simulink model "wlan_phy_rx_pmd"  (Simulink 7.8)
settings: Solver=VariableStepDiscrete  SolverMode=SingleTasking  StartTime=0.0  StopTime=rx_sim.sim_time  RelTol=1e-3
PostLoadFcn:
  | wlan_phy_rx_init
InitFcn:
  | wlan_phy_rx_init
StartFcn:
  | wlan_phy_rx_init
values from neighbouring files (symbols this model uses):
  barker_seq20 = 0.95 * circshift(barker_seq20, [0 4]) ./ max(abs(barker_seq20))    [wlan_phy_rx_init.m]
  bit_scrambler_lfsr_bytes = sum(bit_scrambler_lfsr_bytes .* repmat(2.^[0:7], 127, 1),2)    [wlan_phy_rx_init.m]
  longCorr_coef_i = [3*real(longCorr_coef)]    [wlan_phy_rx_init.m]
  longCorr_coef_q = [3*imag(longCorr_coef)]    [wlan_phy_rx_init.m]
  MAX_CP_LEN = 32    [wlan_phy_rx_init.m]
  MAX_NCBPS = NCBPS_11n_64QAM    (= 312)    [wlan_phy_rx_init.m]
  MAX_NUM_BYTES = 2^16-1    (= 65535)    [wlan_phy_rx_init.m]
  MAX_NUM_SC = 64    [wlan_phy_rx_init.m]
  MAX_NUM_SYMS = 600    [wlan_phy_rx_init.m]
  NDBPS_11a_BPSK12 = 24    [wlan_phy_rx_init.m]
  preFFT_sampBuff_numSamps = 4*MAX_NUM_SC    (= 256)    [wlan_phy_rx_init.m]
  rx_sim = struct()    [wlan_phy_rx_init.m]
  scr_ind_rev = zeros(1,128)    [wlan_phy_rx_init.m]
undefined symbols (not defined in the file or in its folder): gcbh, mcs_rom_11ag, mcs_rom_11n, wlan_rx_deinterleave_rom_gen, xlBlockLoadCallback, xlGetNormalizedPeriod, xlipgetpartsetting
libraries: xbsIndex_r4 x4943, xbsEDKLib_r4 x1
(part 9 of 28)

== system wlan_phy_rx_pmd/Chan Est & EQ/Debug Outputs/4x Buffer ==
  I : Inport
  Q : Inport  Port=2
  Vin : Inport  Port=3
  14LSB : xbsIndex_r4/Slice  nbits=14  boolean_output=off  mode=Lower Bit Location + Width  bit1=0  base1=MSB of Input  bit0=0  base0=LSB of Input  dbl_ovrd=off  block_type=slice
  14MSB : xbsIndex_r4/Slice  nbits=14  boolean_output=off  mode=Upper Bit Location + Width  bit1=0  base1=MSB of Input  bit0=0  base0=LSB of Input  dbl_ovrd=off  block_type=slice
  Concat : xbsIndex_r4/Concat  num_inputs=2  dbl_ovrd=off  block_type=concat
  Constant3 : xbsIndex_r4/Constant  const=1  gui_display_data_type=Boolean  arith_type=Boolean  n_bits=5  bin_pt=0  preci_type=Single  exp_width=8  frac_width=24  explicit_period=on  period=4  equ=P=C  opselect=C  inp2=PCIN>>17  opr=+  inp1=P  carry=CIN  dbl_ovrd=off  block_type=constant
  FIFO : xbsIndex_r4/FIFO  mem_type=Block RAM  performance_options=Standard_FIFO  infoedit1=Available only for V4, V5, V6, V7, K7, A7 and Zynq devices.  embedded_registers=off  depth=64  percent_nbits=1  rst=off  en=off  use_dcount=off  use_percent_full_port=off  use_almost_empty=off  almost_empty_thresh=2  use_almost_full=off  almost_full_thresh=14  dbl_ovrd=off  explicit_period=off  block_type=fifo
  Reinterpret : xbsIndex_r4/Reinterpret  force_arith_type=on  arith_type=Unsigned  force_bin_pt=on  bin_pt=0  block_type=reinterpret
  Reinterpret1 : xbsIndex_r4/Reinterpret  force_arith_type=on  arith_type=Unsigned  force_bin_pt=on  bin_pt=0  block_type=reinterpret
  Reinterpret2 : xbsIndex_r4/Reinterpret  force_arith_type=on  arith_type="Signed  (2's comp)"  force_bin_pt=on  bin_pt=13  block_type=reinterpret
  Reinterpret3 : xbsIndex_r4/Reinterpret  force_arith_type=on  arith_type="Signed  (2's comp)"  force_bin_pt=on  bin_pt=13  block_type=reinterpret
  Up Sample : xbsIndex_r4/Up Sample  sample_ratio=4  copy_samples=on  en=off  latency=0  dbl_ovrd=off  block_type=usamp
  Up Sample1 : xbsIndex_r4/Up Sample  sample_ratio=4  copy_samples=on  en=off  latency=0  dbl_ovrd=off  block_type=usamp
  " I" : Outport
  " Q" : Outport  Port=2
  #Vout : Outport  Port=3
  wires:
  Constant3/1 -> FIFO/3
  Concat/1 -> FIFO/1
  I/1 -> Reinterpret/1
  14MSB/1 -> Reinterpret2/1
  Q/1 -> Reinterpret1/1
  14LSB/1 -> Reinterpret3/1
  Vin/1 -> FIFO/2
  FIFO/2 -> #Vout/1
  Reinterpret/1 -> Concat/1
  Reinterpret1/1 -> Concat/2
  Reinterpret2/1 -> Up Sample/1
  Reinterpret3/1 -> Up Sample1/1
  Up Sample/1 -> " I"/1
  Up Sample1/1 -> " Q"/1
  FIFO/1 -> 14LSB/1, 14MSB/1

== system wlan_phy_rx_pmd/Chan Est & EQ/Equalizer, Pilot Processing & PHY Mode Det/Mag ==
  I : Inport
  Q : Inport  Port=2
  AddSub : xbsIndex_r4/AddSub  mode=Addition  use_carryin=off  use_carryout=off  en=off  latency=4  precision=Full  arith_type=Unsigned  n_bits=16  bin_pt=14  quantization=Truncate  overflow=Wrap  dbl_ovrd=off  use_behavioral_HDL=off  hw_selection=DSP48  pipelined=off  block_type=addsub
  Mult : xbsIndex_r4/Mult  precision=Full  arith_type="Signed  (2's comp)"  n_bits=16  bin_pt=14  quantization=Truncate  overflow=Wrap  en=off  latency=2  dbl_ovrd=off  use_behavioral_HDL=off  opt=Speed  use_embedded=on  optimum_pipeline=off  pipeline=on  use_rpm=on  placement_style=Triangular  block_type=mult
  Mult1 : xbsIndex_r4/Mult  precision=Full  arith_type="Signed  (2's comp)"  n_bits=16  bin_pt=14  quantization=Truncate  overflow=Wrap  en=off  latency=2  dbl_ovrd=off  use_behavioral_HDL=off  opt=Speed  use_embedded=on  optimum_pipeline=off  pipeline=on  use_rpm=on  placement_style=Triangular  block_type=mult
  Register1 : xbsIndex_r4/Register  init=0  rst=off  en=off  dbl_ovrd=off  block_type=register
  Register2 : xbsIndex_r4/Register  init=0  rst=off  en=off  dbl_ovrd=off  block_type=register
  Register3 : xbsIndex_r4/Register  init=0  rst=off  en=off  dbl_ovrd=off  block_type=register
  M : Outport
  wires:
  I/1 -> Mult/1, Mult/2
  Q/1 -> Mult1/1, Mult1/2
  Mult/1 -> Register2/1
  Mult1/1 -> Register1/1
  AddSub/1 -> Register3/1
  Register2/1 -> AddSub/1
  Register1/1 -> AddSub/2
  Register3/1 -> M/1

== system wlan_phy_rx_pmd/Chan Est & EQ/Equalizer, Pilot Processing & PHY Mode Det/PHY Mode Det ==
  note: OFDM Symbols in 11a/g:
      | 0: LTF (BPSK)
      | 1: LTF (BPSK)
      | 2: SIG (BPSK)
      | 3-N: Data
  note: PHY Mode
      | 001: 11a/g
      | 010: 11n
      | 100: 11ac
  note: OFDM Symbols in 11n SISO:
      | 0: L-LTF (BPSK)
      | 1: L-LTF (BPSK)
      | 2: L-SIG (BPSK)
      | 3: HT-SIG1 (QBPSK)
      | 4: HT-SIG2 (QBPSK)
      | 5: HT-STF
      | 6: HT-LFT1
      | 7-N: Data
  note: OFDM Symbols in 11ac SISO:
      | 0: L-LTF (BPSK)
      | 1: L-LTF (BPSK)
      | 2: L-SIG (BPSK)
      | 3: VHT-SIGA1 (BPSK)
      | 4: VHT-SIGA2 (QBPSK)
      | 5: VHT-STF
      | 6: VHT-LFT1
      | 7: VHT-SIGB
      | 8-N: Data
  note: Assert rates here to avoid
      | rate ambiguity when these
      | signals are fed back to earlier
      | blocks in the pipeline
  note: Mark all Rx as 11a/g when 11n mode
      | is disabled.
  x_I : Inport
  x_Q : Inport  Port=2
  x_valid : Inport  Port=3
  Sym ind : Inport  Port=4
  " 11ac" : xbsIndex_r4/Register  init=0  rst=on  en=on  dbl_ovrd=off  block_type=register
  " 11ag" : xbsIndex_r4/Register  init=1  rst=on  en=on  dbl_ovrd=off  block_type=register
  " 11n" : xbsIndex_r4/Register  init=0  rst=on  en=on  dbl_ovrd=off  block_type=register
  Absolute : xbsIndex_r4/Absolute  precision=User Defined  arith_type="Signed  (2's comp)"  n_bits=8  bin_pt=7  quantization=Truncate  overflow=Saturate  en=off  latency=1  dbl_ovrd=off  block_type=abs
  Absolute1 : xbsIndex_r4/Absolute  precision=User Defined  arith_type="Signed  (2's comp)"  n_bits=8  bin_pt=7  quantization=Truncate  overflow=Saturate  en=off  latency=1  dbl_ovrd=off  block_type=abs
  Assert : xbsIndex_r4/Assert  assert_type=off  type_source=Explicitly  gui_display_data_type=Fixed-point  arith_type="Signed  (2's comp)"  n_bits=16  bin_pt=14  preci_type=Single  exp_width=8  frac_width=24  assert_rate=on  rate_source=Explicitly  period=1  output_port=on  block_type=assert
  Assert1 : xbsIndex_r4/Assert  assert_type=off  type_source=Explicitly  gui_display_data_type=Fixed-point  arith_type="Signed  (2's comp)"  n_bits=16  bin_pt=14  preci_type=Single  exp_width=8  frac_width=24  assert_rate=on  rate_source=Explicitly  period=1  output_port=on  block_type=assert
  Assert2 : xbsIndex_r4/Assert  assert_type=off  type_source=Explicitly  gui_display_data_type=Fixed-point  arith_type="Signed  (2's comp)"  n_bits=16  bin_pt=14  preci_type=Single  exp_width=8  frac_width=24  assert_rate=on  rate_source=Explicitly  period=1  output_port=on  block_type=assert
  Concat : xbsIndex_r4/Concat  num_inputs=2  dbl_ovrd=off  block_type=concat
  Concat1 : xbsIndex_r4/Concat  num_inputs=3  dbl_ovrd=off  block_type=concat
  Concat2 : xbsIndex_r4/Concat  num_inputs=3  dbl_ovrd=off  block_type=concat
  Constant1 : xbsIndex_r4/Constant  const=0  gui_display_data_type=Fixed-point  arith_type=Unsigned  n_bits=2  bin_pt=0  preci_type=Single  exp_width=8  frac_width=24  explicit_period=off  period=1  equ=P=C  opselect=C  inp2=PCIN>>17  opr=+  inp1=P  carry=CIN  dbl_ovrd=off  block_type=constant
  Constant3 : xbsIndex_r4/Constant  const=3  gui_display_data_type=Fixed-point  arith_type=Unsigned  n_bits=2  bin_pt=0  preci_type=Single  exp_width=8  frac_width=24  explicit_period=off  period=1  equ=P=C  opselect=C  inp2=PCIN>>17  opr=+  inp1=P  carry=CIN  dbl_ovrd=off  block_type=constant
  Constant4 : xbsIndex_r4/Constant  const=4  gui_display_data_type=Fixed-point  arith_type=Unsigned  n_bits=3  bin_pt=0  preci_type=Single  exp_width=8  frac_width=24  explicit_period=off  period=1  equ=P=C  opselect=C  inp2=PCIN>>17  opr=+  inp1=P  carry=CIN  dbl_ovrd=off  block_type=constant
  Constant5 : xbsIndex_r4/Constant  const=1  gui_display_data_type=Fixed-point  arith_type=Unsigned  n_bits=2  bin_pt=0  preci_type=Single  exp_width=8  frac_width=24  explicit_period=off  period=1  equ=P=C  opselect=C  inp2=PCIN>>17  opr=+  inp1=P  carry=CIN  dbl_ovrd=off  block_type=constant
  Constant7 : xbsIndex_r4/Constant  const=2  gui_display_data_type=Fixed-point  arith_type=Unsigned  n_bits=2  bin_pt=0  preci_type=Single  exp_width=8  frac_width=24  explicit_period=off  period=1  equ=P=C  opselect=C  inp2=PCIN>>17  opr=+  inp1=P  carry=CIN  dbl_ovrd=off  block_type=constant
  Constant8 : xbsIndex_r4/Constant  const=2  gui_display_data_type=Fixed-point  arith_type=Unsigned  n_bits=2  bin_pt=0  preci_type=Single  exp_width=8  frac_width=24  explicit_period=off  period=1  equ=P=C  opselect=C  inp2=PCIN>>17  opr=+  inp1=P  carry=CIN  dbl_ovrd=off  block_type=constant
  Convert : xbsIndex_r4/Convert  gui_display_data_type=Fixed-point  arith_type="Signed  (2's comp)"  n_bits=9  bin_pt=7  float_type=Single  exp_bits=8  fraction_bits=24  quantization=Truncate  overflow=Wrap  en=off  latency=0  dbl_ovrd=off  pipeline=off  block_type=convert
  Convert1 : xbsIndex_r4/Convert  gui_display_data_type=Fixed-point  arith_type="Signed  (2's comp)"  n_bits=9  bin_pt=7  float_type=Single  exp_bits=8  fraction_bits=24  quantization=Truncate  overflow=Wrap  en=off  latency=0  dbl_ovrd=off  pipeline=off  block_type=convert
  Convert2 : xbsIndex_r4/Convert  gui_display_data_type=Boolean  arith_type=Boolean  n_bits=9  bin_pt=7  float_type=Single  exp_bits=8  fraction_bits=24  quantization=Truncate  overflow=Wrap  en=off  latency=0  dbl_ovrd=off  pipeline=off  block_type=convert
  Delay{1..3,5,7} (5 alike) : xbsIndex_r4/Delay  rst=off  infoeditControl=Selection of Reset will increase slice count due to use of real FFs and instead of SRLs  en=off  latency=1  dbl_ovrd=off  reg_retiming=off  block_type=delay
  Gateway Out{1..8} (8 alike) : xbsIndex_r4/Gateway Out  inherit_from_input=off  hdl_port=off  timing_constraint=None  locs_specified=off  LOCs={}  UseAsDAC=off  DACChannel='1'  block_type=gatewayout
  I_Accum : xbsIndex_r4/Accumulator  operation=Add  n_bits=16  overflow=Wrap  scale=1  rst=on  infoeditControl=reset for floating point data type must be asserted for a minimum of 2 cycles  hasbypass=off  en=on  latency=0  dbl_ovrd=off  msb_inp=100  msb=100  lsb=-100  use_behavioral_HDL=on  implementation=Fabric  block_type=accum
  Inverter : xbsIndex_r4/Inverter  en=off  latency=0  dbl_ovrd=off  block_type=inv
  LSB : xbsIndex_r4/Slice  nbits=1  boolean_output=off  mode=Lower Bit Location + Width  bit1=0  base1=MSB of Input  bit0=0  base0=LSB of Input  dbl_ovrd=off  block_type=slice
  Logical1 : xbsIndex_r4/Logical  logical_function=AND  inputs=2  en=off  latency=0  precision=Full  arith_type=Unsigned  n_bits=16  bin_pt=0  align_bp=on  dbl_ovrd=off  block_type=logical
  Logical2 : xbsIndex_r4/Logical  logical_function=AND  inputs=2  en=off  latency=0  precision=Full  arith_type=Unsigned  n_bits=16  bin_pt=0  align_bp=on  dbl_ovrd=off  block_type=logical
  Logical3 : xbsIndex_r4/Logical  logical_function=OR  inputs=2  en=off  latency=0  precision=Full  arith_type=Unsigned  n_bits=16  bin_pt=0  align_bp=on  dbl_ovrd=off  block_type=logical
  Logical4 : xbsIndex_r4/Logical  logical_function=NOR  inputs=2  en=off  latency=0  precision=Full  arith_type=Unsigned  n_bits=16  bin_pt=0  align_bp=on  dbl_ovrd=off  block_type=logical
  Logical5 : xbsIndex_r4/Logical  logical_function=OR  inputs=2  en=off  latency=0  precision=Full  arith_type=Unsigned  n_bits=16  bin_pt=0  align_bp=on  dbl_ovrd=off  block_type=logical
  Mod Det : Subsystem
  Mod_Sym_3 : xbsIndex_r4/Register  init=0  rst=on  en=on  dbl_ovrd=off  block_type=register
  Mod_Sym_4 : xbsIndex_r4/Register  init=0  rst=on  en=on  dbl_ovrd=off  block_type=register
  PHY Mode Det : Scope  NumInputPorts=8
      settings: TimeRange=10000  YMin=-2~-2~0~0~0~0~3.8~0.95  YMax=1.5~1.5~1~6~50~50~4.2~1.05  SaveName=ScopeData11
  Q_Accum : xbsIndex_r4/Accumulator  operation=Add  n_bits=16  overflow=Wrap  scale=1  rst=on  infoeditControl=reset for floating point data type must be asserted for a minimum of 2 cycles  hasbypass=off  en=on  latency=0  dbl_ovrd=off  msb_inp=100  msb=100  lsb=-100  use_behavioral_HDL=on  implementation=Fabric  block_type=accum
  Relational{1..4,6..7} (6 alike) : xbsIndex_r4/Relational  mode=a=b  en=off  latency=0  dbl_ovrd=off  block_type=relational
  edge : Subsystem
  negedge : Subsystem
  negedge1 : Subsystem
  11ac : Outport
  11n : Outport  Port=2
  11a/g : Outport  Port=3
  wires:
  Sym ind/1 -> Relational1/1, Relational2/1, Relational3/1, LSB/1, Gateway Out2/1
  Constant1/1 -> Relational1/2
  Relational1/1 -> Mod_Sym_3/2, Mod_Sym_4/2, " 11ag"/2, " 11n"/2, " 11ac"/2
  Constant3/1 -> Relational2/2
  Constant4/1 -> Relational3/2
  negedge/1(Q) -> Delay3/1
  Relational2/1 -> negedge/1(D)
  Convert/1 -> Absolute/1
  Relational3/1 -> negedge1/1(D)
  negedge1/1(Q) -> Delay5/1
  Convert1/1 -> Absolute1/1
  Absolute/1 -> I_Accum/1
  Absolute1/1 -> Q_Accum/1
  x_valid/1 -> Delay7/1, Gateway Out3/1
  x_I/1 -> Convert/1, Gateway Out1/1
  x_Q/1 -> Convert1/1, Gateway Out4/1
  Delay7/1 -> Q_Accum/3, I_Accum/3
  LSB/1 -> edge/1(D)
  edge/1(Q) -> Convert2/1
  I_Accum/1 -> Mod Det/1(I), Gateway Out7/1
  Q_Accum/1 -> Mod Det/2(Q), Gateway Out8/1
  Concat/1 -> Mod_Sym_3/1, Mod_Sym_4/1
  Constant5/1 -> Relational4/2
  Mod_Sym_3/1 -> Relational4/1, Relational6/1
  Constant7/1 -> Relational6/2
  Mod Det/2(QBPSK) -> Concat/1, Concat2/2
  Mod Det/3(BPSK) -> Concat/2, Concat2/3
  Mod_Sym_4/1 -> Relational7/1
  Constant8/1 -> Relational7/2
  Relational6/1 -> Logical4/2, " 11n"/1
  Relational7/1 -> Logical2/2
  Relational4/1 -> Logical2/1
  Logical2/1 -> Logical4/1, " 11ac"/1
  Logical4/1 -> " 11ag"/1
  Delay3/1 -> Mod_Sym_3/3, Delay1/1
  Delay5/1 -> Mod_Sym_4/3, Delay2/1
  Logical3/1 -> " 11ag"/3, " 11n"/3, " 11ac"/3
  " 11ag"/1 -> Concat1/3, Assert2/1
  Gateway Out1/1 -> PHY Mode Det/1  "X_I"
  Gateway Out4/1 -> PHY Mode Det/2  "X_Q"
  Gateway Out2/1 -> PHY Mode Det/4  "Sym_Ind"
  Gateway Out3/1 -> PHY Mode Det/3  "x_valid"
  Concat2/1 -> Gateway Out5/1
  Gateway Out5/1 -> PHY Mode Det/7  "mod_det"
  Gateway Out6/1 -> PHY Mode Det/8  "PHY_mode"
  Gateway Out7/1 -> PHY Mode Det/5  "I_Accum"
  Gateway Out8/1 -> PHY Mode Det/6  "Q_Accum"
  Convert2/1 -> Q_Accum/2, I_Accum/2
  Mod Det/1(QAM) -> Concat2/1
  " 11n"/1 -> Concat1/2, Assert1/1
  " 11ac"/1 -> Concat1/1, Assert/1
  Concat1/1 -> Gateway Out6/1
  Assert/1 -> 11ac/1
  Assert1/1 -> Logical1/2
  Assert2/1 -> Logical5/1
  Delay2/1 -> Logical3/1
  Delay1/1 -> Logical3/2
  Logical1/1 -> 11n/1
  [regRx_PHY_MODE_11N_ENABLE] -> Logical1/1, Inverter/1
  Inverter/1 -> Logical5/2
  Logical5/1 -> 11a/g/1

== system wlan_phy_rx_pmd/Chan Est & EQ/Equalizer, Pilot Processing & PHY Mode Det/Phase Err Calc ==
  CP_I : Inport
  CP_Q : Inport  Port=2
  x_valid : Inport  Port=3
  x_ind : Inport  Port=4
  sym_ind : Inport  Port=5
  Constant3 : xbsIndex_r4/Constant  const=0  gui_display_data_type=Fixed-point  arith_type=Unsigned  n_bits=6  bin_pt=0  preci_type=Single  exp_width=8  frac_width=24  explicit_period=off  period=8  equ=P=C  opselect=C  inp2=PCIN>>17  opr=+  inp1=P  carry=CIN  dbl_ovrd=off  block_type=constant
  Count : Subsystem
  Delay8 : xbsIndex_r4/Delay  rst=off  infoeditControl=Selection of Reset will increase slice count due to use of real FFs and instead of SRLs  en=off  latency=1  dbl_ovrd=off  reg_retiming=off  block_type=delay
  Descramble : Subsystem
  Gateway Out{1..10} (10 alike) : xbsIndex_r4/Gateway Out  inherit_from_input=off  hdl_port=off  timing_constraint=None  locs_specified=off  LOCs={}  UseAsDAC=off  DACChannel='1'  block_type=gatewayout
  IQ Accum : Subsystem
  Phase Calc : Subsystem
  Pilot Proc : Scope  NumInputPorts=10
      settings: TimeRange=20000  YMin=0~0~0~-0.5~-1~-0.8~-0.1~-0.25~-0.4~-0.4  YMax=80~40~1~1~0~0~0.3~0~0.4~0.4  SaveName=ScopeData10
  Pilot Selection : Subsystem
  Register1 : xbsIndex_r4/Register  init=0  rst=off  en=on  dbl_ovrd=off  block_type=register
  Register2 : xbsIndex_r4/Register  init=0  rst=off  en=on  dbl_ovrd=off  block_type=register
  Relational1 : xbsIndex_r4/Relational  mode=a=b  en=off  latency=0  dbl_ovrd=off  block_type=relational
      settings: xl_area=[2 0 0 3 0 0 0]  block_version=VER_STRING_GOES_HERE
  Relational7 : xbsIndex_r4/Relational  mode=a=b  en=off  latency=0  dbl_ovrd=off  block_type=relational
      settings: xl_area=[2 0 0 3 0 0 0]  block_version=VER_STRING_GOES_HERE
  Sym Phase Err : Outport
  phase err valid : Outport  Port=2
  wires:
  Relational7/1 -> Count/2(Rst), Gateway Out2/1, IQ Accum/4(Rst)
  Descramble/1(" x_I") -> Register1/1, IQ Accum/1(I)
  Descramble/2(" x_Q") -> Register2/1, IQ Accum/2(Q)
  Delay8/1 -> IQ Accum/3(En), Count/1(En), Register1/2, Register2/2
  Count/1(Last) -> Phase Calc/3(IQ Valid)
  Pilot Selection/1(Pilot) -> Delay8/1
  Pilot Selection/2(Negate) -> Descramble/1(Negate)
  CP_I/1 -> Gateway Out9/1, Descramble/2(x_I)
  CP_Q/1 -> Gateway Out10/1, Descramble/3(x_Q)
  Phase Calc/1(phase err) -> Sym Phase Err/1, Gateway Out5/1
  sym_ind/1 -> Relational1/1, Pilot Selection/3(sym_ind), Descramble/4(Sym Ind), Gateway Out1/1
  Relational1/1 -> Phase Calc/4(Reset)
  x_valid/1 -> Pilot Selection/1(x_valid)
  x_ind/1 -> Pilot Selection/2(x_ind), Relational7/1, Gateway Out8/1
  Constant3/1 -> Relational7/2, Relational1/2
  Gateway Out8/1 -> Pilot Proc/1  "Sc Ind"
  Gateway Out3/1 -> Pilot Proc/4  "Accum I"
  Gateway Out4/1 -> Pilot Proc/5  "Accum Q"
  Gateway Out5/1 -> Pilot Proc/6  "Phase Err"
  Gateway Out6/1 -> Pilot Proc/7  "Descram I"
  Gateway Out7/1 -> Pilot Proc/8  "Descram Q"
  Gateway Out9/1 -> Pilot Proc/9  "Conj Prod I"
  Gateway Out10/1 -> Pilot Proc/10  "Conj Prod Q"
  Register1/1 -> Gateway Out6/1
  Register2/1 -> Gateway Out7/1
  Gateway Out1/1 -> Pilot Proc/2  "Sym Ind"
  Gateway Out2/1 -> Pilot Proc/3  "Accum Resets"
  IQ Accum/1(I Sum) -> Phase Calc/1(I), Gateway Out3/1
  IQ Accum/2(Q Sum) -> Phase Calc/2(Q), Gateway Out4/1
  Phase Calc/2(valid) -> phase err valid/1

== system wlan_phy_rx_pmd/Chan Est & EQ/Equalizer, Pilot Processing & PHY Mode Det/a*conj(b) ==
  note: Implements A*conj(B)
  A_I : Inport
  A_Q : Inport  Port=2
  B_I : Inport  Port=3
  B_Q : Inport  Port=4
  AddSub : xbsIndex_r4/AddSub  mode=Addition  use_carryin=off  use_carryout=off  en=off  latency=4  precision=Full  arith_type=Unsigned  n_bits=16  bin_pt=14  quantization=Truncate  overflow=Wrap  dbl_ovrd=off  use_behavioral_HDL=off  hw_selection=DSP48  pipelined=off  block_type=addsub
  AddSub1 : xbsIndex_r4/AddSub  mode=Subtraction  use_carryin=off  use_carryout=off  en=off  latency=4  precision=Full  arith_type=Unsigned  n_bits=16  bin_pt=14  quantization=Truncate  overflow=Wrap  dbl_ovrd=off  use_behavioral_HDL=off  hw_selection=DSP48  pipelined=off  block_type=addsub
  Mult{1..4} (4 alike) : xbsIndex_r4/Mult  precision=Full  arith_type="Signed  (2's comp)"  n_bits=16  bin_pt=14  quantization=Truncate  overflow=Wrap  en=off  latency=2  dbl_ovrd=off  use_behavioral_HDL=off  opt=Speed  use_embedded=on  optimum_pipeline=off  pipeline=on  use_rpm=on  placement_style=Triangular  block_type=mult
  Register{1..6} (6 alike) : xbsIndex_r4/Register  init=0  rst=off  en=off  dbl_ovrd=off  block_type=register
  P_I : Outport
  P_Q : Outport  Port=2
  wires:
  A_I/1 -> Mult1/1, Mult4/2
  B_I/1 -> Mult1/2, Mult3/2
  A_Q/1 -> Mult2/1, Mult3/1
  B_Q/1 -> Mult2/2, Mult4/1
  Mult1/1 -> Register2/1
  Mult2/1 -> Register1/1
  Mult3/1 -> Register3/1
  Mult4/1 -> Register4/1
  AddSub/1 -> Register5/1
  AddSub1/1 -> Register6/1
  Register2/1 -> AddSub/1
  Register1/1 -> AddSub/2
  Register3/1 -> AddSub1/1
  Register4/1 -> AddSub1/2
  Register5/1 -> P_I/1
  Register6/1 -> P_Q/1

== system wlan_phy_rx_pmd/Chan Est & EQ/Phase Tracking & Correction/Bool Safe ==
  note: Simulation-only block: Sets output to 0
      | if input is indeterminate, which occurs at
      | the blackbox outputs before the first reset
  A : Inport
  Convert1 : xbsIndex_r4/Convert  gui_display_data_type=Fixed-point  arith_type="Signed  (2's comp)"  n_bits=18  bin_pt=14  float_type=Single  exp_bits=8  fraction_bits=24  quantization=Truncate  overflow=Wrap  en=off  latency=0  dbl_ovrd=off  pipeline=off  block_type=convert
  Indet Check : Subsystem
  Simulation Multiplexer : xbsIndex_r4/Simulation Multiplexer  sim_sel=1  hw_sel=2  block_type=simmux
      settings: infoedit="Distinguish input subsystems as \"simulation only\" and \"generation only\".  The input specified For Simulation will be used during Simulink simulation.  The input specified For Generation will be used during code generation.  This block will typically be used on the output of a HW Co-Simulation, Black Box, or ModelSim subsystem.<br><br>Hardware Notes: This block costs nothing."
  B : Outport
  wires:
  A/1 -> Indet Check/1(In1), Simulation Multiplexer/2
  Indet Check/1(Out1) -> Simulation Multiplexer/1
  Simulation Multiplexer/1 -> Convert1/1
  Convert1/1 -> B/1

== system wlan_phy_rx_pmd/Chan Est & EQ/Phase Tracking & Correction/Bool Safe1 ==
  note: Simulation-only block: Sets output to 0
      | if input is indeterminate, which occurs at
      | the blackbox outputs before the first reset
  A : Inport
  Convert1 : xbsIndex_r4/Convert  gui_display_data_type=Fixed-point  arith_type="Signed  (2's comp)"  n_bits=18  bin_pt=14  float_type=Single  exp_bits=8  fraction_bits=24  quantization=Truncate  overflow=Wrap  en=off  latency=0  dbl_ovrd=off  pipeline=off  block_type=convert
  Indet Check : Subsystem
  Simulation Multiplexer : xbsIndex_r4/Simulation Multiplexer  sim_sel=1  hw_sel=2  block_type=simmux
      settings: infoedit="Distinguish input subsystems as \"simulation only\" and \"generation only\".  The input specified For Simulation will be used during Simulink simulation.  The input specified For Generation will be used during code generation.  This block will typically be used on the output of a HW Co-Simulation, Black Box, or ModelSim subsystem.<br><br>Hardware Notes: This block costs nothing."
  B : Outport
  wires:
  Convert1/1 -> B/1
  Simulation Multiplexer/1 -> Convert1/1
  Indet Check/1(Out1) -> Simulation Multiplexer/1
  A/1 -> Simulation Multiplexer/2, Indet Check/1(In1)

== system wlan_phy_rx_pmd/Chan Est & EQ/Phase Tracking & Correction/Phase Rotation ==
  note: Upstream logic pulses Phase Valid for 1 cycle each
      | time the Phase value is updated. However the DDS
      | only outputs valid sin/cos values when the phase_tvalid
      | signal is asserted. Since the complex mult will use the
      | I/Q tvalid signal to qualify the output tvalid, we can
      | safely leave the DDS phase_tvalid input asserted all the time.
  x_I : Inport
  x_Q : Inport  Port=2
  x_valid : Inport  Port=3
  Phase : Inport  Port=4
  Phase Valid : Inport  Port=5
  "x_ind " : Inport  Port=6
  "sym_ind " : Inport  Port=7
  Bus Creator : BusCreator  Inputs=2
  "Complex Multiplier 5.0 " : xbsIndex_r4/Complex Multiplier 5.0   hasatlast=off  hasatuser=off  atuserwidth=1  hasbtlast=off  hasbtuser=off  btuserwidth=1  multtype=Use_Mults  optimizegoal=Resources  flowcontrol=NonBlocking  outputwidth=18  roundmode=Truncate  hasctrltlast=off  hasctrltuser=off  ctrltuserwidth=1  outtlastbehv=Null  latencyconfig=Automatic  minimumlatency=37  aclken=off  aresetn=off  trim_axipin_name=on  aportwidth=63  bportwidth=63  ip_name=Complex Multiplier  ip_version=5.0  dsptool_ready=true  ipcore_usecache=true  ipcore_useipmodelcache=true  ipcore_verbose=false  ipcore_latency_parameter='minimumlatency'  wrapper_available=true  port_translation_map={ 'aclken' => 'en', 'aresetn' => 'rst'}  structural_sim=false  block_type=cmpy_v5_0
  Constant1 : xbsIndex_r4/Constant  const=1  gui_display_data_type=Boolean  arith_type=Boolean  n_bits=16  bin_pt=13  preci_type=Single  exp_width=8  frac_width=24  explicit_period=on  period=1  equ=P=C  opselect=C  inp2=PCIN>>17  opr=+  inp1=P  carry=CIN  dbl_ovrd=off  block_type=constant
  Constant2 : xbsIndex_r4/Constant  const=1  gui_display_data_type=Boolean  arith_type=Boolean  n_bits=16  bin_pt=13  preci_type=Single  exp_width=8  frac_width=24  explicit_period=on  period=1  equ=P=C  opselect=C  inp2=PCIN>>17  opr=+  inp1=P  carry=CIN  dbl_ovrd=off  block_type=constant
  Constant3 : xbsIndex_r4/Constant  const=1  gui_display_data_type=Fixed-point  arith_type=Signed (2's comp)  n_bits=16  bin_pt=15  preci_type=Single  exp_width=8  frac_width=24  explicit_period=on  period=1  equ=P=C  opselect=C  inp2=PCIN>>17  opr=+  inp1=P  carry=CIN  dbl_ovrd=off  block_type=constant
  Constant4 : xbsIndex_r4/Constant  const=0  gui_display_data_type=Fixed-point  arith_type=Signed (2's comp)  n_bits=16  bin_pt=15  preci_type=Single  exp_width=8  frac_width=24  explicit_period=on  period=1  equ=P=C  opselect=C  inp2=PCIN>>17  opr=+  inp1=P  carry=CIN  dbl_ovrd=off  block_type=constant
  Convert : xbsIndex_r4/Convert  gui_display_data_type=Fixed-point  arith_type="Signed  (2's comp)"  n_bits=16  bin_pt=15  float_type=Single  exp_bits=8  fraction_bits=24  quantization=Truncate  overflow=Wrap  en=off  latency=0  dbl_ovrd=off  pipeline=off  block_type=convert
  "DDS Compiler 5.0 " : xbsIndex_r4/DDS Compiler 5.0   partspresent=SIN_COS_LUT_only  dds_clock_rate=100  channels=1  parameter_entry=Hardware_Parameters  spurious_free_dynamic_range=36  frequency_resolution=0.4  noise_shaping=None  phase_width=16  output_width=16  output_selection=Sine_and_Cosine  negative_sine=on  negative_cosine=off  amplitude_mode=Full_Range  memory_type=Auto  optimization_goal=Auto  dsp48_use=Minimal  latency_configuration=Auto  latency=8  has_phase_out=off  has_aclken=off  has_aresetn=off  explicit_period=off  period=1  data_has_tlast=Not_Required  has_tready=on  s_phase_has_tuser=Not_Required  m_data_has_tuser=Not_Required  m_phase_has_tuser=Not_Required  s_phase_tuser_width=1  s_config_sync_mode=On_Vector  phase_increment=Fixed  output_frequency1=0  output_frequency2=0  output_frequency3=0  output_frequency4=0  output_frequency5=0  output_frequency6=0  output_frequency7=0  output_frequency8=0  output_frequency9=0  output_frequency10=0  output_frequency11=0  output_frequency12=0  output_frequency13=0  output_frequency14=0  output_frequency15=0  output_frequency16=0  pinc1='0'  pinc2='0'  pinc3='0'  pinc4='0'  pinc5='0'  pinc6='0'  pinc7='0'  pinc8='0'  pinc9='0'  pinc10='0'  pinc11='0'  pinc12='0'  pinc13='0'  pinc14='0'  pinc15='0'  pinc16='0'  phase_offset=None  phase_offset_angles1=0  phase_offset_angles2=0  phase_offset_angles3=0  phase_offset_angles4=0  phase_offset_angles5=0  phase_offset_angles6=0  phase_offset_angles7=0  phase_offset_angles8=0  phase_offset_angles9=0  phase_offset_angles10=0  phase_offset_angles11=0  phase_offset_angles12=0  phase_offset_angles13=0  phase_offset_angles14=0  phase_offset_angles15=0  phase_offset_angles16=0  poff1='0'  poff2='0'  poff3='0'  poff4='0'  poff5='0'  poff6='0'  poff7='0'  poff8='0'  poff9='0'  poff10='0'  poff11='0'  poff12='0'  poff13='0'  poff14='0'  poff15='0'  poff16='0'  trim_axipin_name=on  por_mode=false  gui_behaviour=Sysgen  ip_name=DDS Compiler  ip_version=5.0  dsptool_ready=true  wrapper_available=true  port_translation_map={ 'aclken' => 'en', 'aresetn' => 'rst' }  ipcore_xco_need_fpga_part=true  ipcore_fpga_part=xlipgetpartsetting(gcb, {'virtex6', 'xc6vlx75t', '-3', 'ff784'})  ipcore_usecache=true  ipcore_useipmodelcache=true  ipcore_verbose=false  block_type=dds_compiler_v5_0
  Delay1 : xbsIndex_r4/Delay  rst=off  infoeditControl=Selection of Reset will increase slice count due to use of real FFs and instead of SRLs  en=off  latency=6  dbl_ovrd=off  reg_retiming=off  block_type=delay
  Delay18 : xbsIndex_r4/Delay  rst=off  infoeditControl=Selection of Reset will increase slice count due to use of real FFs and instead of SRLs  en=off  latency=6  dbl_ovrd=off  reg_retiming=off  block_type=delay
  Delay{2..6} (5 alike) : xbsIndex_r4/Delay  rst=off  infoeditControl=Selection of Reset will increase slice count due to use of real FFs and instead of SRLs  en=off  latency=12  dbl_ovrd=off  reg_retiming=off  block_type=delay
  Gateway Out{1,3..6,8..13} (11 alike) : xbsIndex_r4/Gateway Out  inherit_from_input=off  hdl_port=off  timing_constraint=None  locs_specified=off  LOCs={}  UseAsDAC=off  DACChannel='1'  block_type=gatewayout
  Phase Correct : Scope  NumInputPorts=10
      settings: TimeRange=50000  YMin=0~-2~-2~0~-0.8~0~-2~-3~-1~0  YMax=1~2~2~2~0~1~3~2~1~1  SaveName=ScopeData14
  " x_I" : Outport
  " x_Q" : Outport  Port=2
  " x_valid" : Outport  Port=3
  x_ind : Outport  Port=4
  " sym ind" : Outport  Port=5
  wires:
  Gateway Out12/1 -> Phase Correct/5  "Phase"
  Gateway Out11/1 -> Phase Correct/3  "X_Q"
  Gateway Out9/1 -> Phase Correct/7  "I Out"
  Gateway Out8/1 -> Phase Correct/2  "X_I"
  Gateway Out5/1 -> Phase Correct/1  "X_IQ Valid"
  Gateway Out13/1 -> Phase Correct/6  "Valid Out"
  Gateway Out10/1 -> Phase Correct/8  "Q Out"
  Delay1/1 -> x_ind/1
  Delay18/1 -> " sym ind"/1
  Phase/1 -> Convert/1
  Constant1/1 -> "DDS Compiler 5.0 "/3
  Constant2/1 -> "DDS Compiler 5.0 "/1, Gateway Out1/1
  x_valid/1 -> Delay6/1
  x_Q/1 -> Delay5/1
  x_I/1 -> Delay4/1
  "DDS Compiler 5.0 "/2 -> "Complex Multiplier 5.0 "/4, Gateway Out3/1
  "DDS Compiler 5.0 "/3 -> Gateway Out6/1, "Complex Multiplier 5.0 "/5
  "DDS Compiler 5.0 "/4 -> Gateway Out4/1, "Complex Multiplier 5.0 "/6
  "Complex Multiplier 5.0 "/1 -> " x_valid"/1, Gateway Out13/1
  "Complex Multiplier 5.0 "/2 -> " x_Q"/1, Gateway Out10/1
  "Complex Multiplier 5.0 "/3 -> " x_I"/1, Gateway Out9/1
  "sym_ind "/1 -> Delay2/1
  "x_ind "/1 -> Delay3/1
  Gateway Out1/1 -> Phase Correct/4  "Phase valid"
  Bus Creator/1 -> Phase Correct/9  "Sin-Cos"
  Gateway Out3/1 -> Phase Correct/10  "Sin-Cos Valid"
  Convert/1 -> "DDS Compiler 5.0 "/2, Gateway Out12/1
  Gateway Out4/1 -> Bus Creator/1
  Gateway Out6/1 -> Bus Creator/2
  Delay2/1 -> Delay18/1
  Delay3/1 -> Delay1/1
  Delay4/1 -> "Complex Multiplier 5.0 "/3, Gateway Out8/1
  Delay5/1 -> "Complex Multiplier 5.0 "/2, Gateway Out11/1
  Delay6/1 -> "Complex Multiplier 5.0 "/1, Gateway Out5/1

== system wlan_phy_rx_pmd/DSSS Rx/Demod/Bit-to-Byte ==
  Bit : Inport
  Bit Valid : Inport  Port=2
  Concat : xbsIndex_r4/Concat  num_inputs=8  dbl_ovrd=off  block_type=concat
  Constant : xbsIndex_r4/Constant  const=8  gui_display_data_type=Fixed-point  arith_type=Unsigned  n_bits=4  bin_pt=0  preci_type=Single  exp_width=8  frac_width=24  explicit_period=off  period=1  equ=P=C  opselect=C  inp2=PCIN>>17  opr=+  inp1=P  carry=CIN  dbl_ovrd=off  block_type=constant
  Counter : xbsIndex_r4/Counter  cnt_type=Free Running  cnt_to=Inf  operation=Up  start_count=0  cnt_by_val=1  arith_type=Unsigned  n_bits=8  bin_pt=0  load_pin=off  rst=on  en=on  explicit_period=off  period=1  dbl_ovrd=off  use_behavioral_HDL=off  implementation=Fabric  block_type=counter
  Counter1 : xbsIndex_r4/Counter  cnt_type=Free Running  cnt_to=Inf  operation=Up  start_count=0  cnt_by_val=1  arith_type=Unsigned  n_bits=ceil(log2(MAX_NUM_BYTES))  bin_pt=0  load_pin=off  rst=on  en=on  explicit_period=off  period=1  dbl_ovrd=off  use_behavioral_HDL=off  implementation=Fabric  block_type=counter
  Delay : xbsIndex_r4/Delay  rst=off  infoeditControl=Selection of Reset will increase slice count due to use of real FFs and instead of SRLs  en=off  latency=1  dbl_ovrd=off  reg_retiming=off  block_type=delay
  Delay1 : xbsIndex_r4/Delay  rst=off  infoeditControl=Selection of Reset will increase slice count due to use of real FFs and instead of SRLs  en=off  latency=1  dbl_ovrd=off  reg_retiming=off  block_type=delay
  Logical1 : xbsIndex_r4/Logical  logical_function=OR  inputs=2  en=off  latency=0  precision=Full  arith_type=Unsigned  n_bits=8  bin_pt=2  align_bp=on  dbl_ovrd=off  block_type=logical
      settings: xl_area=[1 0 0 1 0 0 0]  block_version=VER_STRING_GOES_HERE
  Register{,1..8} (9 alike) : xbsIndex_r4/Register  init=0  rst=on  en=on  dbl_ovrd=off  block_type=register
  Relational : xbsIndex_r4/Relational  mode=a=b  en=off  latency=0  dbl_ovrd=off  block_type=relational
  Byte : Outport
  Byte Valid : Outport  Port=2
  Byte Ind : Outport  Port=3
  wires:
  Register/1 -> Register1/1, Concat/1
  Register1/1 -> Register2/1, Concat/2
  Register2/1 -> Register3/1, Concat/3
  Register3/1 -> Register4/1, Concat/4
  Register4/1 -> Register5/1, Concat/5
  Register5/1 -> Register6/1, Concat/6
  Register6/1 -> Register7/1, Concat/7
  Bit Valid/1 -> Register1/3, Register2/3, Register3/3, Register4/3, Register5/3, Register6/3, Register7/3, Register/3, Counter/2
  [DSSS_RX_RESET] -> Register6/2, Register7/2, Register5/2, Register4/2, Register3/2, Register2/2, Register1/2, Register/2, Logical1/2
  Bit/1 -> Register/1
  Register7/1 -> Concat/8
  Concat/1 -> Register8/1
  Constant/1 -> Relational/2
  Counter/1 -> Relational/1
  Logical1/1 -> Counter/1
  Relational/1 -> Logical1/1, Register8/3, Delay1/1, Delay/1
  [DSSS_RX_RESET] -> Register8/2
  Register8/1 -> Byte/1
  Delay/1 -> Byte Valid/1
  [DSSS_RX_RESET] -> Counter1/1
  Counter1/1 -> Byte Ind/1
  Delay1/1 -> Counter1/2

== system wlan_phy_rx_pmd/DSSS Rx/Demod/DBPSK Demod ==
  IQ Valid : Inport
  I : Inport  Port=2
  Q : Inport  Port=3
  Absolute : xbsIndex_r4/Absolute  precision=Full  arith_type=Unsigned  n_bits=16  bin_pt=14  quantization=Truncate  overflow=Wrap  en=off  latency=0  dbl_ovrd=off  block_type=abs
  AddSub : xbsIndex_r4/AddSub  mode=Subtraction  use_carryin=off  use_carryout=off  en=off  latency=0  precision=Full  arith_type=Unsigned  n_bits=16  bin_pt=14  quantization=Truncate  overflow=Wrap  dbl_ovrd=off  use_behavioral_HDL=off  hw_selection=Fabric  pipelined=off  block_type=addsub
  "CORDIC 5.0 " : xbsIndex_r4/CORDIC 5.0   functional_selection=Arc_Tan  architectural_configuration=Parallel  pipelining_mode=Maximum  data_format=SignedFraction  phase_format=Scaled_Radians  input_width=16  output_width=16  round_mode=Truncate  iterations=0  precision=0  compensation_scaling=No_Scale_Compensation  coarse_rotation=on  aclken=off  aresetn=off  out_tready=off  cartesian_has_tuser=off  cartesian_has_tlast=off  cartesian_tuser_width=1  phase_has_tuser=off  phase_has_tlast=off  phase_tuser_width=1  out_tlast_behv=Null  flow_control=NonBlocking  optimize_goal=Resources  trim_axipin_name=on  ip_name=CORDIC  ip_version=5.0  dsptool_ready=true  ipcore_usecache=true  ipcore_useipmodelcache=true  wrapper_available=true  port_translation_map={ 'aclken' => 'en', 'aresetn' => 'rst' }  block_type=cordic_v5_0
      settings: infoedit=CORDIC 5.0
  Constant : xbsIndex_r4/Constant  const=0.5  gui_display_data_type=Fixed-point  arith_type=Signed (2's comp)  n_bits=4  bin_pt=3  preci_type=Single  exp_width=8  frac_width=24  explicit_period=off  period=1  equ=P=C  opselect=C  inp2=PCIN>>17  opr=+  inp1=P  carry=CIN  dbl_ovrd=off  block_type=constant
  Constant1 : xbsIndex_r4/Constant  const=1.5  gui_display_data_type=Fixed-point  arith_type=Signed (2's comp)  n_bits=6  bin_pt=3  preci_type=Single  exp_width=8  frac_width=24  explicit_period=off  period=1  equ=P=C  opselect=C  inp2=PCIN>>17  opr=+  inp1=P  carry=CIN  dbl_ovrd=off  block_type=constant
  Convert : xbsIndex_r4/Convert  gui_display_data_type=Fixed-point  arith_type=Unsigned  n_bits=1  bin_pt=0  float_type=Single  exp_bits=8  fraction_bits=24  quantization=Truncate  overflow=Wrap  en=off  latency=0  dbl_ovrd=off  pipeline=off  block_type=convert
  Gateway Out2 : xbsIndex_r4/Gateway Out  inherit_from_input=off  hdl_port=off  timing_constraint=None  locs_specified=off  LOCs={}  UseAsDAC=off  DACChannel='1'  block_type=gatewayout
  Gateway Out3 : xbsIndex_r4/Gateway Out  inherit_from_input=off  hdl_port=off  timing_constraint=None  locs_specified=off  LOCs={}  UseAsDAC=off  DACChannel='1'  block_type=gatewayout
  Gateway Out6 : xbsIndex_r4/Gateway Out  inherit_from_input=off  hdl_port=off  timing_constraint=None  locs_specified=off  LOCs={}  UseAsDAC=off  DACChannel='1'  block_type=gatewayout
  Logical : xbsIndex_r4/Logical  logical_function=AND  inputs=2  en=off  latency=0  precision=Full  arith_type=Unsigned  n_bits=16  bin_pt=0  align_bp=on  dbl_ovrd=off  block_type=logical
  Phase Cmp : Scope  NumInputPorts=7
      settings: TimeRange=80000  YMin=-1~-0.5~0~-0.1~5~0~0  YMax=1~0.3~1~1.1~40~1~300  SaveName=ScopeData6
  Register{,1..3} (4 alike) : xbsIndex_r4/Register  init=0  rst=off  en=off  dbl_ovrd=off  block_type=register
  Register4 : xbsIndex_r4/Register  init=0  rst=off  en=on  dbl_ovrd=off  block_type=register
  Relational : xbsIndex_r4/Relational  mode=a>b  en=off  latency=0  dbl_ovrd=off  block_type=relational
  Relational1 : xbsIndex_r4/Relational  mode=a<b  en=off  latency=0  dbl_ovrd=off  block_type=relational
  bit : Outport
  bit valid : Outport  Port=2
  wires:
  IQ Valid/1 -> "CORDIC 5.0 "/1
  I/1 -> "CORDIC 5.0 "/2
  Q/1 -> "CORDIC 5.0 "/3
  "CORDIC 5.0 "/2 -> Register1/1
  "CORDIC 5.0 "/1 -> Register/1
  Register4/1 -> AddSub/2, Gateway Out3/1
  AddSub/1 -> Absolute/1
  Constant/1 -> Relational/2
  Absolute/1 -> Gateway Out6/1, Relational/1, Relational1/1
  Relational/1 -> Logical/1
  Convert/1 -> Register2/1
  Gateway Out2/1 -> Phase Cmp/1
  Gateway Out3/1 -> Phase Cmp/2
  Gateway Out6/1 -> Phase Cmp/3
  Constant1/1 -> Relational1/2
  Relational1/1 -> Logical/2
  Logical/1 -> Convert/1
  Register/1 -> Register3/1, Register4/2
  Register1/1 -> AddSub/1, Gateway Out2/1, Register4/1
  Register2/1 -> bit/1
  Register3/1 -> bit valid/1

== system wlan_phy_rx_pmd/DSSS Rx/Demod/De-spread ==
  I : Inport
  Q : Inport  Port=2
  Sym Start : Inport  Port=3
  Accumulator : xbsIndex_r4/Accumulator  operation=Add  n_bits=22  overflow=Wrap  scale=1  rst=on  infoeditControl=reset for floating point data type must be asserted for a minimum of 2 cycles  hasbypass=on  en=off  latency=0  dbl_ovrd=off  msb_inp=100  msb=100  lsb=-100  use_behavioral_HDL=on  implementation=Fabric  block_type=accum
  Accumulator1 : xbsIndex_r4/Accumulator  operation=Add  n_bits=22  overflow=Wrap  scale=1  rst=on  infoeditControl=reset for floating point data type must be asserted for a minimum of 2 cycles  hasbypass=on  en=off  latency=0  dbl_ovrd=off  msb_inp=100  msb=100  lsb=-100  use_behavioral_HDL=on  implementation=Fabric  block_type=accum
  Constant : xbsIndex_r4/Constant  const=0  gui_display_data_type=Fixed-point  arith_type=Unsigned  n_bits=2  bin_pt=0  preci_type=Single  exp_width=8  frac_width=24  explicit_period=off  period=1  equ=P=C  opselect=C  inp2=PCIN>>17  opr=+  inp1=P  carry=CIN  dbl_ovrd=off  block_type=constant
  Constant1 : xbsIndex_r4/Constant  const=0  gui_display_data_type=Boolean  arith_type=Boolean  n_bits=16  bin_pt=14  preci_type=Single  exp_width=8  frac_width=24  explicit_period=on  period=8  equ=P=C  opselect=C  inp2=PCIN>>17  opr=+  inp1=P  carry=CIN  dbl_ovrd=off  block_type=constant
  Convert : xbsIndex_r4/Convert  gui_display_data_type=Fixed-point  arith_type="Signed  (2's comp)"  n_bits=16  bin_pt=14  float_type=Single  exp_bits=8  fraction_bits=24  quantization=Truncate  overflow=Saturate  en=off  latency=0  dbl_ovrd=off  pipeline=off  block_type=convert
  Convert1 : xbsIndex_r4/Convert  gui_display_data_type=Fixed-point  arith_type="Signed  (2's comp)"  n_bits=16  bin_pt=14  float_type=Single  exp_bits=8  fraction_bits=24  quantization=Truncate  overflow=Saturate  en=off  latency=0  dbl_ovrd=off  pipeline=off  block_type=convert
  Counter : xbsIndex_r4/Counter  cnt_type=Count Limited  cnt_to=19  operation=Up  start_count=0  cnt_by_val=1  arith_type=Unsigned  n_bits=5  bin_pt=0  load_pin=off  rst=on  en=on  explicit_period=off  period=1  dbl_ovrd=off  use_behavioral_HDL=off  implementation=Fabric  block_type=counter
  Delay : xbsIndex_r4/Delay  rst=off  infoeditControl=Selection of Reset will increase slice count due to use of real FFs and instead of SRLs  en=off  latency=1  dbl_ovrd=off  reg_retiming=off  block_type=delay
  Gateway Out{1..9} (9 alike) : xbsIndex_r4/Gateway Out  inherit_from_input=off  hdl_port=off  timing_constraint=None  locs_specified=off  LOCs={}  UseAsDAC=off  DACChannel='1'  block_type=gatewayout
  Inverter : xbsIndex_r4/Inverter  en=off  latency=0  dbl_ovrd=off  block_type=inv
  Logical1 : xbsIndex_r4/Logical  logical_function=AND  inputs=2  en=off  latency=0  precision=Full  arith_type=Unsigned  n_bits=8  bin_pt=2  align_bp=on  dbl_ovrd=off  block_type=logical
      settings: xl_area=[1 0 0 1 0 0 0]  block_version=VER_STRING_GOES_HERE
  Mult : xbsIndex_r4/Mult  precision=User Defined  arith_type="Signed  (2's comp)"  n_bits=16  bin_pt=15  quantization=Truncate  overflow=Wrap  en=off  latency=1  dbl_ovrd=off  use_behavioral_HDL=off  opt=Speed  use_embedded=on  optimum_pipeline=off  pipeline=on  use_rpm=on  placement_style=Triangular  block_type=mult
  Mult1 : xbsIndex_r4/Mult  precision=User Defined  arith_type="Signed  (2's comp)"  n_bits=16  bin_pt=15  quantization=Truncate  overflow=Wrap  en=off  latency=1  dbl_ovrd=off  use_behavioral_HDL=off  opt=Speed  use_embedded=on  optimum_pipeline=off  pipeline=on  use_rpm=on  placement_style=Triangular  block_type=mult
  ROM : xbsIndex_r4/ROM  depth=length(barker_seq20)  initVector=fliplr(barker_seq20)  distributed_mem=Block RAM  rst=off  init_reg=0  en=off  latency=1  gui_display_data_type=Fixed-point  arith_type="Signed  (2's comp)"  n_bits=16  bin_pt=15  preci_type=Single  exp_width=8  frac_width=24  dbl_ovrd=off  optimize=Area  use_rpm=on  block_type=sprom
  Real-Imag to Complex : RealImagToComplex
  Register : xbsIndex_r4/Register  init=0  rst=on  en=on  dbl_ovrd=off  block_type=register
  Register1 : xbsIndex_r4/Register  init=0  rst=on  en=on  dbl_ovrd=off  block_type=register
  Register2 : xbsIndex_r4/Register  init=0  rst=on  en=off  dbl_ovrd=off  block_type=register
  Register3 : xbsIndex_r4/Register  init=0  rst=on  en=off  dbl_ovrd=off  block_type=register
  Register{4..7} (4 alike) : xbsIndex_r4/Register  init=0  rst=off  en=off  dbl_ovrd=off  block_type=register
  Relational : xbsIndex_r4/Relational  mode=a=b  en=off  latency=0  dbl_ovrd=off  block_type=relational
  Scope : Scope  NumInputPorts=7
      settings: TimeRange=60000  YMin=-1~-1~-1~-1~-0.4~-0.5~0  YMax=1~1~0.5~1~0.4~1~1  SaveName=ScopeData4
  To Workspace : ToWorkspace  VariableName=simout  SampleTime=-1  SaveFormat=Array
  IQ Valid : Outport
  " I" : Outport  Port=2
  " Q" : Outport  Port=3
  tag scope: CS_DSSS_Despread_I=global, CS_DSSS_Despread_Q=global
  wires:
  I/1 -> Register4/1
  Counter/1 -> ROM/1, Relational/1
  ROM/1 -> Register6/1
  Mult/1 -> Accumulator/1
  Mult1/1 -> Accumulator1/1
  Constant/1 -> Relational/2
  Relational/1 -> Register7/1
  Inverter/1 -> Counter/1, Register3/2, Register2/2, Register/2, Register1/2
  Accumulator/1 -> Register/1, Gateway Out8/1
  Accumulator1/1 -> Register1/1, Gateway Out9/1
  Real-Imag to Complex/1 -> To Workspace/1
  Register2/1 -> Gateway Out2/1
  Register3/1 -> Gateway Out3/1
  Gateway Out2/1 -> Real-Imag to Complex/1
  Gateway Out3/1 -> Real-Imag to Complex/2
  Register/1 -> Convert/1, Gateway Out1/1
  Q/1 -> Register5/1
  Register1/1 -> Convert1/1, Gateway Out6/1
  Delay/1 -> Logical1/1
  Sym Start/1 -> Logical1/2, Inverter/1, Gateway Out7/1, Counter/2
  Convert/1 -> " I"/1, [CS_DSSS_Despread_I]
  Convert1/1 -> " Q"/1, [CS_DSSS_Despread_Q]
  Logical1/1 -> IQ Valid/1
  Gateway Out4/1 -> Scope/1
  Gateway Out5/1 -> Scope/2
  Gateway Out8/1 -> Scope/3
  Gateway Out9/1 -> Scope/4
  Gateway Out1/1 -> Scope/5
  Gateway Out6/1 -> Scope/6
  Register4/1 -> Mult/1, Register2/1, Gateway Out4/1
  Register5/1 -> Mult1/1
  Register6/1 -> Mult1/2, Mult/2, Register3/1, Gateway Out5/1
  Register7/1 -> Accumulator1/2, Register1/3, Register/3, Delay/1, Accumulator/2
  Gateway Out7/1 -> Scope/7

== system wlan_phy_rx_pmd/DSSS Rx/Demod/Descramble ==
  Bit : Inport
  Bit Valid : Inport  Port=2
  Convert : xbsIndex_r4/Convert  gui_display_data_type=Boolean  arith_type=Boolean  n_bits=16  bin_pt=14  float_type=Single  exp_bits=8  fraction_bits=24  quantization=Truncate  overflow=Wrap  en=off  latency=0  dbl_ovrd=off  pipeline=off  block_type=convert
  Convert1 : xbsIndex_r4/Convert  gui_display_data_type=Boolean  arith_type=Boolean  n_bits=16  bin_pt=14  float_type=Single  exp_bits=8  fraction_bits=24  quantization=Truncate  overflow=Wrap  en=off  latency=0  dbl_ovrd=off  pipeline=off  block_type=convert
  Convert2 : xbsIndex_r4/Convert  gui_display_data_type=Fixed-point  arith_type=Unsigned  n_bits=1  bin_pt=0  float_type=Single  exp_bits=8  fraction_bits=24  quantization=Truncate  overflow=Wrap  en=off  latency=0  dbl_ovrd=off  pipeline=off  block_type=convert
  Logical : xbsIndex_r4/Logical  logical_function=XOR  inputs=2  en=off  latency=0  precision=Full  arith_type=Unsigned  n_bits=8  bin_pt=2  align_bp=on  dbl_ovrd=off  block_type=logical
      settings: xl_area=[0, 0, 0, 0, 0, 0, 0]  block_version=VER_STRING_GOES_HERE
  Logical1 : xbsIndex_r4/Logical  logical_function=XOR  inputs=2  en=off  latency=0  precision=Full  arith_type=Unsigned  n_bits=8  bin_pt=2  align_bp=on  dbl_ovrd=off  block_type=logical
      settings: xl_area=[0, 0, 0, 0, 0, 0, 0]  block_version=VER_STRING_GOES_HERE
  Register{,1..6} (7 alike) : xbsIndex_r4/Register  init=1  rst=on  en=on  dbl_ovrd=off  block_type=register
      settings: xl_area=[0, 0, 0, 0, 0, 0, 0]  block_version=VER_STRING_GOES_HERE
  Register7 : xbsIndex_r4/Register  init=0  rst=off  en=off  dbl_ovrd=off  block_type=register
  Register8 : xbsIndex_r4/Register  init=0  rst=off  en=off  dbl_ovrd=off  block_type=register
  " Bit" : Outport
  " Bit Valid" : Outport  Port=2
  wires:
  [DSSS_RX_RESET] -> Convert/1
  Register6/1 -> Logical/1
  Register5/1 -> Register6/1
  Register4/1 -> Register5/1
  Register3/1 -> Register4/1, Logical/2
  Register2/1 -> Register3/1
  Register1/1 -> Register2/1
  Register/1 -> Register1/1
  Bit Valid/1 -> Convert1/1
  Convert/1 -> Register5/2, Register6/2, Register4/2, Register3/2, Register2/2, Register1/2, Register/2
  Convert1/1 -> Register5/3, Register6/3, Register8/1, Register4/3, Register3/3, Register2/3, Register1/3, Register/3
  Convert2/1 -> Register7/1
  Bit/1 -> Register/1, Logical1/1
  Logical/1 -> Logical1/2
  Logical1/1 -> Convert2/1
  Register7/1 -> " Bit"/1
  Register8/1 -> " Bit Valid"/1

== system wlan_phy_rx_pmd/DSSS Rx/Demod/Header CRC ==
  Bit : Inport
  Bit Valid : Inport  Port=2
  CRC LFSR : Subsystem
  Constant : xbsIndex_r4/Constant  const=32  gui_display_data_type=Fixed-point  arith_type=Unsigned  n_bits=6  bin_pt=0  preci_type=Single  exp_width=8  frac_width=24  explicit_period=off  period=1  equ=P=C  opselect=C  inp2=PCIN>>17  opr=+  inp1=P  carry=CIN  dbl_ovrd=off  block_type=constant
  Counter1 : xbsIndex_r4/Counter  cnt_type=Free Running  cnt_to=Inf  operation=Up  start_count=0  cnt_by_val=1  arith_type=Unsigned  n_bits=8  bin_pt=0  load_pin=off  rst=on  en=on  explicit_period=off  period=1  dbl_ovrd=off  use_behavioral_HDL=off  implementation=Fabric  block_type=counter
  Display : Display
  Gateway Out{1..6} (6 alike) : xbsIndex_r4/Gateway Out  inherit_from_input=off  hdl_port=off  timing_constraint=None  locs_specified=off  LOCs={}  UseAsDAC=off  DACChannel='1'  block_type=gatewayout
  Logical1 : xbsIndex_r4/Logical  logical_function=AND  inputs=2  en=off  latency=0  precision=Full  arith_type=Unsigned  n_bits=16  bin_pt=0  align_bp=on  dbl_ovrd=off  block_type=logical
  Register8 : xbsIndex_r4/Register  init=0  rst=off  en=on  dbl_ovrd=off  block_type=register
  Relational1 : xbsIndex_r4/Relational  mode=a<b  en=off  latency=0  dbl_ovrd=off  block_type=relational
  Scope : Scope  NumInputPorts=7
      settings: TimeRange=100000  YMin=-1~-1~-1~-0.5~0~0~0  YMax=1~1~1~1.5~150~1~1  SaveName=ScopeData3
  CRC Calc : Outport
  wires:
  [DSSS_RX_RESET] -> Counter1/1, Gateway Out6/1
  Counter1/1 -> Relational1/1, Gateway Out2/1
  Constant/1 -> Relational1/2
  Relational1/1 -> Logical1/2
  Bit/1 -> CRC LFSR/1(Bit), Register8/1
  CRC LFSR/1(CRC16) -> Gateway Out4/1, CRC Calc/1
  Gateway Out4/1 -> Display/1
  Gateway Out1/1 -> Scope/6
  Gateway Out5/1 -> Scope/7
  Gateway Out2/1 -> Scope/5
  Gateway Out3/1 -> Scope/4
  Register8/1 -> Gateway Out3/1
  Gateway Out6/1 -> Scope/1
  Bit Valid/1 -> Logical1/1, Gateway Out1/1
  Logical1/1 -> Counter1/2, CRC LFSR/2(Bit Valid), Gateway Out5/1, Register8/2
